simulink model "ofdm_txrx_mimo_2x"  (Simulink 6.6)
settings: Solver=VariableStepDiscrete  StartTime=0.0  StopTime=20e3  RelTol=1e-3
PreLoadFcn:
  | ofdm_rx_mimo_init
InitFcn:
  | ofdm_rx_mimo_init
StartFcn:
  | ofdm_rx_mimo_init
values from neighbouring files (symbols this model uses):
  BER_RAM_init_size = 2048    [ofdm_tx_mimo_init.m]
  CPLength = 16    [ofdm_tx_mimo_init.m]
  CRCPolynomial = hex2dec('04c11db7')    [ofdm_tx_mimo_init.m]
  freqOffset_track_filtCoef_i = hex2dec('2500')/2^32    [ofdm_rx_mimo_init.m]
  freqOffset_track_filtCoef_p = hex2dec('d0000')/2^32    [ofdm_rx_mimo_init.m]
  hi = zeros(1,length(cci))    [ofdm_rx_mimo_init.m]
  hr = zeros(1,length(ccr))    [ofdm_rx_mimo_init.m]
  max_CP_length = 16    [ofdm_tx_mimo_init.m]
  max_num_baseRateSymbols = 31    [ofdm_tx_mimo_init.m]
  max_num_subcarriers = 64    [ofdm_tx_mimo_init.m]
  max_num_trainingSymbols = 15    [ofdm_tx_mimo_init.m]
  max_numBytes = 16384    [ofdm_tx_mimo_init.m]
  max_OFDM_symbols = 2047    [ofdm_tx_mimo_init.m]
  modConstellation_qam16 = .75*[1 3 -1 -3]./3    [ofdm_tx_mimo_init.m]
  modConstellation_qam256 = 0.9375*[3 1 5 7 11 9 13 15 -3 -1 -5 -7 -11 -9 -13 -15]./15    [ofdm_tx_mimo_init.m]
  modConstellation_qam64 = 0.875*[3 1 5 7 -3 -1 -5 -7]./7    [ofdm_tx_mimo_init.m]
  modConstellation_qpsk = [1 -1]./sqrt(2)    [ofdm_tx_mimo_init.m]
  numBaseRateSymbols = 2    [ofdm_tx_mimo_init.m]
  numSubcarriers = 64    [ofdm_tx_mimo_init.m]
  numTrainingSymbols = 2    [ofdm_tx_mimo_init.m]
  pilot1_indicies = 7 + ( (64-7) * 2^16)    (= 3735559)    [ofdm_tx_mimo_init.m]
  pilot2_indicies = 21 + ( (64-21) * 2^16)    (= 2818069)    [ofdm_tx_mimo_init.m]
  pilotValue_neg = hex2dec('8000') + (2^16 * 0)    [ofdm_tx_mimo_init.m]
  pilotValue_pos = hex2dec('7FFF') + (2^16 * 0)    [ofdm_tx_mimo_init.m]
  pkt_crossCorr_thresh = 0.7    [ofdm_rx_mimo_init.m]
  pkt_energy_thresh = 0    [ofdm_rx_mimo_init.m]
  pkt_numPayloadBytes = 1400+42-6    (= 1436)    [ofdm_tx_mimo_init.m]
  pktByteNums = numHeaderBytes +  				byteIndex_numPayloadBytes(1)*2^8 +  				byteIndex_numPayloadBytes(2)*2^16 +  				byteIndex_simpleDynModMasks * 2^24    [ofdm_rx_mimo_init.m]
  PktDet_Delay = 26 + Symbol_Timing_Offset*2^7    (= 1050)    [ofdm_rx_mimo_init.m]
  preamble = 6*[0 shortsyms_10 longsyms_2]    [ofdm_tx_mimo_init.m]
  preamble_I = real(preamble)    [ofdm_tx_mimo_init.m]
  preamble_Q = imag(preamble)    [ofdm_tx_mimo_init.m]
  RAM_init_size = max_numBytes    (= 16384)    [ofdm_tx_mimo_init.m]
  reg_Interrupt_PktBuf_Control = reg_InterruptControl +  	1 * 2^16 +  	0 * 2^24    (= 65656)    [ofdm_rx_mimo_init.m]
  rx_controlBits = 1 * 2  		 + 1 * 4  		 + 0 * 8  		 + 1 * 16 * rx_SISO_Mode  		 + 1 * 32  		 + 1 * 64  		 + 0 * 128  		 + 1 * 256  		 + 0 * 512  		 + 1 * 1024  		 + 0 * 2048  		 + 1 * 4096  		 + 1 * 8192  		 + 0 * 16384  		 + 1 * 65536  		 + 1 * 131072  		 + 0 * 262144  		 + 1 * 524288    [ofdm_rx_mimo_init.m]
  Rx_PhaseNoiseTrack_K = 0.6094    [ofdm_rx_mimo_init.m]
  rx_postEq_scaling = round(rxScaling*2^11) * (1 + 2^16)    [ofdm_rx_mimo_init.m]
  RxDataScrambling_Seq = [40 198 78 63 82 173 102 245 48 111 172 115 147 230 216 93 72 65 62 2 205 242 122 90 128 83 105 97 73 10 5 252]    [ofdm_tx_mimo_init.m]
  simOnly_numSamples = length(preamble)+( (numSubcarriers+CPLength)*(numTrainingSymbols + numBaseRateSymbols + numFullRateSymbols/2) )    [ofdm_tx_mimo_init.m]
  train = [train train]    [ofdm_tx_mimo_init.m]
  tx_controlBits = (antB_preambleShift * 2^4) + (2^0 * tx_SISO_Mode) + 4*0 + 8*1    (= 73)    [ofdm_tx_mimo_init.m]
  TxDataScrambling_Seq = [40 198 78 63 82 173 102 245 48 111 172 115 147 230 216 93 72 65 62 2 205 242 122 90 128 83 105 97 73 10 5 252]    [ofdm_tx_mimo_init.m]
  txReg_symbolCounts = (2^16 * numFullRateSymbols) + (2^8 *numBaseRateSymbols ) + numTrainingSymbols    [ofdm_tx_mimo_init.m]
  TxRx_FFTScaling = bin2dec('010111') + (bin2dec('000101') * 2^6)    [ofdm_tx_mimo_init.m]
undefined symbols (not defined in the file or in its folder): AntA, AntB, BaseRate, CRC_table_gen, gcbh, intfilt, pktTiming_count_payloadStart, pktTiming_symbol_length, xlBlockLoadCallback, xlGetSimulinkPeriod
libraries: xbsIndex_r4 x3973, dspsigops x4, commchan3 x2, commrflib2 x2, dspsrcs4 x1
(part 7 of 38)

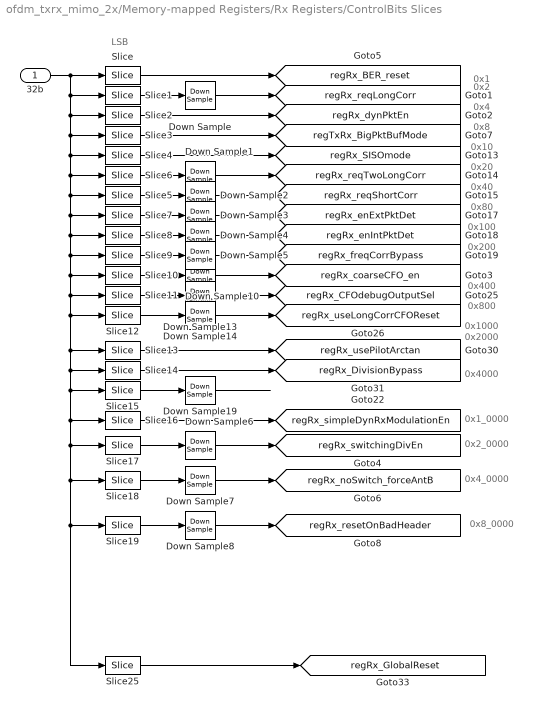
== system ofdm_txrx_mimo_2x/Memory-mapped Registers/Rx Registers/ControlBits Slices ==
  note: LSB
  note: 0x1
  note: 0x2
  note: 0x4
  note: 0x8
  note: 0x10
  note: 0x20
  note: 0x40
  note: 0x80
  note: 0x100
  note: 0x200
  note: 0x400
  note: 0x800
  note: 0x1000
  note: 0x2000
  note: 0x4000
  note: 0x1_0000
  note: 0x2_0000
  note: 0x4_0000
  note: 0x8_0000
  32b : Inport
  Down Sample{,1..8,10,13..14,19} (13 alike) : xbsIndex_r4/Down Sample  sample_ratio=2  sample_phase="Last Value of Frame  (most efficient)"  en=off  latency=1  dbl_ovrd=off  block_type=dsamp
      settings: block_version=VER_STRING_GOES_HERE
  Slice : xbsIndex_r4/Slice  nbits=1  boolean_output=on  mode=Lower Bit Location + Width  bit1=0  base1=MSB of Input  bit0=0  base0=LSB of Input  dbl_ovrd=off  block_type=slice
      settings: infoedit="Extracts a given range of bits from each input sample and presents it at the output.  The output type is ordinarily unsigned with binary point at zero, but can be Boolean when the slice is one bit wide.<P><P>Hardware notes: In hardware this block costs nothing."  block_version=VER_STRING_GOES_HERE
  Slice1 : xbsIndex_r4/Slice  nbits=1  boolean_output=on  mode=Lower Bit Location + Width  bit1=0  base1=MSB of Input  bit0=1  base0=LSB of Input  dbl_ovrd=off  block_type=slice
      settings: infoedit="Extracts a given range of bits from each input sample and presents it at the output.  The output type is ordinarily unsigned with binary point at zero, but can be Boolean when the slice is one bit wide.<P><P>Hardware notes: In hardware this block costs nothing."  block_version=VER_STRING_GOES_HERE
  Slice10 : xbsIndex_r4/Slice  nbits=1  boolean_output=on  mode=Lower Bit Location + Width  bit1=0  base1=MSB of Input  bit0=10  base0=LSB of Input  dbl_ovrd=off  block_type=slice
      settings: infoedit="Extracts a given range of bits from each input sample and presents it at the output.  The output type is ordinarily unsigned with binary point at zero, but can be Boolean when the slice is one bit wide.<P><P>Hardware notes: In hardware this block costs nothing."  block_version=VER_STRING_GOES_HERE
  Slice11 : xbsIndex_r4/Slice  nbits=1  boolean_output=on  mode=Lower Bit Location + Width  bit1=0  base1=MSB of Input  bit0=11  base0=LSB of Input  dbl_ovrd=off  block_type=slice
      settings: infoedit="Extracts a given range of bits from each input sample and presents it at the output.  The output type is ordinarily unsigned with binary point at zero, but can be Boolean when the slice is one bit wide.<P><P>Hardware notes: In hardware this block costs nothing."  block_version=VER_STRING_GOES_HERE
  Slice12 : xbsIndex_r4/Slice  nbits=1  boolean_output=on  mode=Lower Bit Location + Width  bit1=0  base1=MSB of Input  bit0=12  base0=LSB of Input  dbl_ovrd=off  block_type=slice
      settings: infoedit="Extracts a given range of bits from each input sample and presents it at the output.  The output type is ordinarily unsigned with binary point at zero, but can be Boolean when the slice is one bit wide.<P><P>Hardware notes: In hardware this block costs nothing."  block_version=VER_STRING_GOES_HERE
  Slice13 : xbsIndex_r4/Slice  nbits=1  boolean_output=on  mode=Lower Bit Location + Width  bit1=0  base1=MSB of Input  bit0=13  base0=LSB of Input  dbl_ovrd=off  block_type=slice
      settings: infoedit="Extracts a given range of bits from each input sample and presents it at the output.  The output type is ordinarily unsigned with binary point at zero, but can be Boolean when the slice is one bit wide.<P><P>Hardware notes: In hardware this block costs nothing."  block_version=VER_STRING_GOES_HERE
  Slice14 : xbsIndex_r4/Slice  nbits=1  boolean_output=on  mode=Lower Bit Location + Width  bit1=0  base1=MSB of Input  bit0=14  base0=LSB of Input  dbl_ovrd=off  block_type=slice
      settings: infoedit="Extracts a given range of bits from each input sample and presents it at the output.  The output type is ordinarily unsigned with binary point at zero, but can be Boolean when the slice is one bit wide.<P><P>Hardware notes: In hardware this block costs nothing."  block_version=VER_STRING_GOES_HERE
  Slice15 : xbsIndex_r4/Slice  nbits=1  boolean_output=on  mode=Lower Bit Location + Width  bit1=0  base1=MSB of Input  bit0=15  base0=LSB of Input  dbl_ovrd=off  block_type=slice
      settings: infoedit="Extracts a given range of bits from each input sample and presents it at the output.  The output type is ordinarily unsigned with binary point at zero, but can be Boolean when the slice is one bit wide.<P><P>Hardware notes: In hardware this block costs nothing."  block_version=VER_STRING_GOES_HERE
  Slice16 : xbsIndex_r4/Slice  nbits=1  boolean_output=on  mode=Lower Bit Location + Width  bit1=0  base1=MSB of Input  bit0=16  base0=LSB of Input  dbl_ovrd=off  block_type=slice
      settings: infoedit="Extracts a given range of bits from each input sample and presents it at the output.  The output type is ordinarily unsigned with binary point at zero, but can be Boolean when the slice is one bit wide.<P><P>Hardware notes: In hardware this block costs nothing."  block_version=VER_STRING_GOES_HERE
  Slice17 : xbsIndex_r4/Slice  nbits=1  boolean_output=on  mode=Lower Bit Location + Width  bit1=0  base1=MSB of Input  bit0=17  base0=LSB of Input  dbl_ovrd=off  block_type=slice
      settings: infoedit="Extracts a given range of bits from each input sample and presents it at the output.  The output type is ordinarily unsigned with binary point at zero, but can be Boolean when the slice is one bit wide.<P><P>Hardware notes: In hardware this block costs nothing."  block_version=VER_STRING_GOES_HERE
  Slice18 : xbsIndex_r4/Slice  nbits=1  boolean_output=on  mode=Lower Bit Location + Width  bit1=0  base1=MSB of Input  bit0=18  base0=LSB of Input  dbl_ovrd=off  block_type=slice
      settings: infoedit="Extracts a given range of bits from each input sample and presents it at the output.  The output type is ordinarily unsigned with binary point at zero, but can be Boolean when the slice is one bit wide.<P><P>Hardware notes: In hardware this block costs nothing."  block_version=VER_STRING_GOES_HERE
  Slice19 : xbsIndex_r4/Slice  nbits=1  boolean_output=on  mode=Lower Bit Location + Width  bit1=0  base1=MSB of Input  bit0=19  base0=LSB of Input  dbl_ovrd=off  block_type=slice
      settings: infoedit="Extracts a given range of bits from each input sample and presents it at the output.  The output type is ordinarily unsigned with binary point at zero, but can be Boolean when the slice is one bit wide.<P><P>Hardware notes: In hardware this block costs nothing."  block_version=VER_STRING_GOES_HERE
  Slice2 : xbsIndex_r4/Slice  nbits=1  boolean_output=on  mode=Lower Bit Location + Width  bit1=0  base1=MSB of Input  bit0=2  base0=LSB of Input  dbl_ovrd=off  block_type=slice
      settings: infoedit="Extracts a given range of bits from each input sample and presents it at the output.  The output type is ordinarily unsigned with binary point at zero, but can be Boolean when the slice is one bit wide.<P><P>Hardware notes: In hardware this block costs nothing."  block_version=VER_STRING_GOES_HERE
  Slice25 : xbsIndex_r4/Slice  nbits=1  boolean_output=on  mode=Upper Bit Location + Width  bit1=0  base1=MSB of Input  bit0=31  base0=LSB of Input  dbl_ovrd=off  block_type=slice
      settings: infoedit="Extracts a given range of bits from each input sample and presents it at the output.  The output type is ordinarily unsigned with binary point at zero, but can be Boolean when the slice is one bit wide.<P><P>Hardware notes: In hardware this block costs nothing."  block_version=VER_STRING_GOES_HERE
  Slice3 : xbsIndex_r4/Slice  nbits=1  boolean_output=on  mode=Lower Bit Location + Width  bit1=0  base1=MSB of Input  bit0=3  base0=LSB of Input  dbl_ovrd=off  block_type=slice
      settings: infoedit="Extracts a given range of bits from each input sample and presents it at the output.  The output type is ordinarily unsigned with binary point at zero, but can be Boolean when the slice is one bit wide.<P><P>Hardware notes: In hardware this block costs nothing."  block_version=VER_STRING_GOES_HERE
  Slice4 : xbsIndex_r4/Slice  nbits=1  boolean_output=on  mode=Lower Bit Location + Width  bit1=0  base1=MSB of Input  bit0=4  base0=LSB of Input  dbl_ovrd=off  block_type=slice
      settings: infoedit="Extracts a given range of bits from each input sample and presents it at the output.  The output type is ordinarily unsigned with binary point at zero, but can be Boolean when the slice is one bit wide.<P><P>Hardware notes: In hardware this block costs nothing."  block_version=VER_STRING_GOES_HERE
  Slice5 : xbsIndex_r4/Slice  nbits=1  boolean_output=on  mode=Lower Bit Location + Width  bit1=0  base1=MSB of Input  bit0=6  base0=LSB of Input  dbl_ovrd=off  block_type=slice
      settings: infoedit="Extracts a given range of bits from each input sample and presents it at the output.  The output type is ordinarily unsigned with binary point at zero, but can be Boolean when the slice is one bit wide.<P><P>Hardware notes: In hardware this block costs nothing."  block_version=VER_STRING_GOES_HERE
  Slice6 : xbsIndex_r4/Slice  nbits=1  boolean_output=on  mode=Lower Bit Location + Width  bit1=0  base1=MSB of Input  bit0=5  base0=LSB of Input  dbl_ovrd=off  block_type=slice
      settings: infoedit="Extracts a given range of bits from each input sample and presents it at the output.  The output type is ordinarily unsigned with binary point at zero, but can be Boolean when the slice is one bit wide.<P><P>Hardware notes: In hardware this block costs nothing."  block_version=VER_STRING_GOES_HERE
  Slice7 : xbsIndex_r4/Slice  nbits=1  boolean_output=on  mode=Lower Bit Location + Width  bit1=0  base1=MSB of Input  bit0=7  base0=LSB of Input  dbl_ovrd=off  block_type=slice
      settings: infoedit="Extracts a given range of bits from each input sample and presents it at the output.  The output type is ordinarily unsigned with binary point at zero, but can be Boolean when the slice is one bit wide.<P><P>Hardware notes: In hardware this block costs nothing."  block_version=VER_STRING_GOES_HERE
  Slice8 : xbsIndex_r4/Slice  nbits=1  boolean_output=on  mode=Lower Bit Location + Width  bit1=0  base1=MSB of Input  bit0=8  base0=LSB of Input  dbl_ovrd=off  block_type=slice
      settings: infoedit="Extracts a given range of bits from each input sample and presents it at the output.  The output type is ordinarily unsigned with binary point at zero, but can be Boolean when the slice is one bit wide.<P><P>Hardware notes: In hardware this block costs nothing."  block_version=VER_STRING_GOES_HERE
  Slice9 : xbsIndex_r4/Slice  nbits=1  boolean_output=on  mode=Lower Bit Location + Width  bit1=0  base1=MSB of Input  bit0=9  base0=LSB of Input  dbl_ovrd=off  block_type=slice
      settings: infoedit="Extracts a given range of bits from each input sample and presents it at the output.  The output type is ordinarily unsigned with binary point at zero, but can be Boolean when the slice is one bit wide.<P><P>Hardware notes: In hardware this block costs nothing."  block_version=VER_STRING_GOES_HERE
  tag scope: regRx_BER_reset=global, regRx_CFOdebugOutputSel=global, regRx_DivisionBypass=global, regRx_GlobalReset=global, regRx_SISOmode=global, regRx_coarseCFO_en=global, regRx_dynPktEn=global, regRx_enExtPktDet=global, regRx_enIntPktDet=global, regRx_freqCorrBypass=global, regRx_noSwitch_forceAntB=global, regRx_reqLongCorr=global, regRx_reqShortCorr=global, regRx_reqTwoLongCorr=global, regRx_resetOnBadHeader=global, regRx_simpleDynRxModulationEn=global, regRx_switchingDivEn=global, regRx_useLongCorrCFOReset=global, regRx_usePilotArctan=global, regTxRx_BigPktBufMode=global
  wires:
  Slice/1 -> [regRx_BER_reset]
  Slice1/1 -> Down Sample/1
  Slice2/1 -> [regRx_dynPktEn]
  Down Sample/1 -> [regRx_reqLongCorr]
  Slice4/1 -> [regRx_SISOmode]
  Slice6/1 -> Down Sample1/1
  Slice5/1 -> Down Sample2/1
  Down Sample1/1 -> [regRx_reqTwoLongCorr]
  Down Sample2/1 -> [regRx_reqShortCorr]
  Slice7/1 -> Down Sample3/1
  Slice8/1 -> Down Sample4/1
  Down Sample3/1 -> [regRx_enExtPktDet]
  Down Sample4/1 -> [regRx_enIntPktDet]
  Slice9/1 -> Down Sample5/1
  Down Sample5/1 -> [regRx_freqCorrBypass]
  32b/1 -> Slice16/1, Slice17/1, Slice18/1, Slice25/1, Slice19/1, Slice15/1, Slice14/1, Slice13/1, Slice12/1, Slice11/1, Slice10/1, Slice9/1, Slice8/1, Slice7/1, Slice5/1, Slice6/1, Slice4/1, Slice3/1, Slice2/1, Slice1/1, Slice/1
  Slice10/1 -> Down Sample10/1
  Down Sample10/1 -> [regRx_coarseCFO_en]
  Slice11/1 -> Down Sample13/1
  Down Sample13/1 -> [regRx_CFOdebugOutputSel]
  Down Sample14/1 -> [regRx_useLongCorrCFOReset]
  Slice12/1 -> Down Sample14/1
  Slice3/1 -> [regTxRx_BigPktBufMode]
  Slice13/1 -> [regRx_usePilotArctan]
  Slice14/1 -> [regRx_DivisionBypass]
  Slice15/1 -> Down Sample19/1
  Down Sample19/1 -> ?
  Slice25/1 -> [regRx_GlobalReset]
  Slice16/1 -> [regRx_simpleDynRxModulationEn]
  Slice17/1 -> Down Sample6/1
  Down Sample6/1 -> [regRx_switchingDivEn]
  Slice18/1 -> Down Sample7/1
  Down Sample7/1 -> [regRx_noSwitch_forceAntB]
  Down Sample8/1 -> [regRx_resetOnBadHeader]
  Slice19/1 -> Down Sample8/1

== system ofdm_txrx_mimo_2x/Memory-mapped Registers/Rx Registers/Read Only Register ==
  D : Inport
  En : Inport  Port=2
  Convert : xbsIndex_r4/Convert  arith_type=Unsigned  n_bits=32  bin_pt=0  quantization=Truncate  overflow=Wrap  latency=0  dbl_ovrd=off  pipeline=off  block_type=convert
      settings: block_version=10.1
  Reinterpret : xbsIndex_r4/Reinterpret  force_arith_type=on  arith_type=Unsigned  force_bin_pt=on  bin_pt=0  block_type=reinterpret
      settings: infoedit="Changes signal type without altering the binary representation.   You can changed the signal between signed and unsigned, and relocate the binary point.<P><P>Hardware notes: In hardware this block costs nothing.<P><P>Example:  Suppose the input is 6 bits wide, signed, with 2 fractional bits, and the output is forced to unsigned with 0 fractional bits.  Then an input of -2.0 (1110.00 in binary 2's complement) becomes an output of 56 (111000 in binary)."  block_version=10.1
  Terminator : Terminator
  To Register : xbsIndex_r4/To Register  shared_memory_name='Rx_Gains'  init=0  ownership=Locally owned and initialized  explicit_data_type=on  arith_type=Unsigned  n_bits=32  bin_pt=0  dbl_ovrd=off  block_type=toreg
      settings: AttributesFormatString=To Register\n<< %<shared_memory_name> >>  infoedit="Register block that writes data to a shared memory register.  Delay of one sample period."  block_version=8.2.02
  wires:
  En/1 -> To Register/2
  To Register/1 -> Terminator/1
  D/1 -> Reinterpret/1
  Reinterpret/1 -> Convert/1
  Convert/1 -> To Register/1

== system ofdm_txrx_mimo_2x/Memory-mapped Registers/Rx Registers/Read Only Register1 ==
  D : Inport
  En : Inport  Port=2
  Convert : xbsIndex_r4/Convert  arith_type=Unsigned  n_bits=32  bin_pt=0  quantization=Truncate  overflow=Wrap  latency=0  dbl_ovrd=off  pipeline=off  block_type=convert
      settings: block_version=10.1
  Reinterpret : xbsIndex_r4/Reinterpret  force_arith_type=on  arith_type=Unsigned  force_bin_pt=on  bin_pt=0  block_type=reinterpret
      settings: infoedit="Changes signal type without altering the binary representation.   You can changed the signal between signed and unsigned, and relocate the binary point.<P><P>Hardware notes: In hardware this block costs nothing.<P><P>Example:  Suppose the input is 6 bits wide, signed, with 2 fractional bits, and the output is forced to unsigned with 0 fractional bits.  Then an input of -2.0 (1110.00 in binary 2's complement) becomes an output of 56 (111000 in binary)."  block_version=10.1
  Terminator : Terminator
  To Register : xbsIndex_r4/To Register  shared_memory_name='Rx_BER_Errors'  init=0  ownership=Locally owned and initialized  explicit_data_type=on  arith_type=Unsigned  n_bits=32  bin_pt=0  dbl_ovrd=off  block_type=toreg
      settings: AttributesFormatString=To Register\n<< %<shared_memory_name> >>  infoedit="Register block that writes data to a shared memory register.  Delay of one sample period."  block_version=8.2.02
  wires:
  En/1 -> To Register/2
  To Register/1 -> Terminator/1
  D/1 -> Reinterpret/1
  Reinterpret/1 -> Convert/1
  Convert/1 -> To Register/1

== system ofdm_txrx_mimo_2x/Memory-mapped Registers/Rx Registers/Read Only Register2 ==
  D : Inport
  En : Inport  Port=2
  Convert : xbsIndex_r4/Convert  arith_type=Unsigned  n_bits=32  bin_pt=0  quantization=Truncate  overflow=Wrap  latency=0  dbl_ovrd=off  pipeline=off  block_type=convert
      settings: block_version=10.1
  Reinterpret : xbsIndex_r4/Reinterpret  force_arith_type=on  arith_type=Unsigned  force_bin_pt=on  bin_pt=0  block_type=reinterpret
      settings: infoedit="Changes signal type without altering the binary representation.   You can changed the signal between signed and unsigned, and relocate the binary point.<P><P>Hardware notes: In hardware this block costs nothing.<P><P>Example:  Suppose the input is 6 bits wide, signed, with 2 fractional bits, and the output is forced to unsigned with 0 fractional bits.  Then an input of -2.0 (1110.00 in binary 2's complement) becomes an output of 56 (111000 in binary)."  block_version=10.1
  Terminator : Terminator
  To Register : xbsIndex_r4/To Register  shared_memory_name='Rx_BER_TotalBits'  init=0  ownership=Locally owned and initialized  explicit_data_type=on  arith_type=Unsigned  n_bits=32  bin_pt=0  dbl_ovrd=off  block_type=toreg
      settings: AttributesFormatString=To Register\n<< %<shared_memory_name> >>  infoedit="Register block that writes data to a shared memory register.  Delay of one sample period."  block_version=8.2.02
  wires:
  En/1 -> To Register/2
  To Register/1 -> Terminator/1
  D/1 -> Reinterpret/1
  Reinterpret/1 -> Convert/1
  Convert/1 -> To Register/1

== system ofdm_txrx_mimo_2x/Memory-mapped Registers/Rx Registers/Read Only Register3 ==
  D : Inport
  En : Inport  Port=2
  Convert : xbsIndex_r4/Convert  arith_type=Unsigned  n_bits=32  bin_pt=0  quantization=Truncate  overflow=Wrap  latency=0  dbl_ovrd=off  pipeline=off  block_type=convert
      settings: block_version=10.1
  Reinterpret : xbsIndex_r4/Reinterpret  force_arith_type=on  arith_type=Unsigned  force_bin_pt=on  bin_pt=0  block_type=reinterpret
      settings: infoedit="Changes signal type without altering the binary representation.   You can changed the signal between signed and unsigned, and relocate the binary point.<P><P>Hardware notes: In hardware this block costs nothing.<P><P>Example:  Suppose the input is 6 bits wide, signed, with 2 fractional bits, and the output is forced to unsigned with 0 fractional bits.  Then an input of -2.0 (1110.00 in binary 2's complement) becomes an output of 56 (111000 in binary)."  block_version=10.1
  Terminator : Terminator
  To Register : xbsIndex_r4/To Register  shared_memory_name='Rx_pktDone_interruptStatus'  init=0  ownership=Locally owned and initialized  explicit_data_type=on  arith_type=Unsigned  n_bits=32  bin_pt=0  dbl_ovrd=off  block_type=toreg
      settings: AttributesFormatString=To Register\n<< %<shared_memory_name> >>  infoedit="Register block that writes data to a shared memory register.  Delay of one sample period."  block_version=8.2.02
  wires:
  En/1 -> To Register/2
  To Register/1 -> Terminator/1
  D/1 -> Reinterpret/1
  Reinterpret/1 -> Convert/1
  Convert/1 -> To Register/1

== system ofdm_txrx_mimo_2x/Memory-mapped Registers/Rx Registers/Read-Write Register ==
  Constant : xbsIndex_r4/Constant  arith_type=Boolean  const=1  n_bits=16  bin_pt=14  explicit_period=on  period=sampPeriod  equ=P=C  opselect=C  inp2=PCIN>>17  opr=+  inp1=P  carry=CIN  dbl_ovrd=off  block_type=constant
      settings: block_version=8.2.02
  From Register : xbsIndex_r4/From Register  shared_memory_name='Rx_ControlBits_w'  init=734566  period=sampPeriod  ownership=Locally owned and initialized  arith_type=Unsigned  n_bits=32  bin_pt=0  dbl_ovrd=off  block_type=fromreg
      settings: AttributesFormatString=From Register\n<< %<shared_memory_name> >>\n%<n_bits>\n%<period>\n%<bin_pt>\n%<arith_type>\n%<ownership>\n%<init>  infoedit="Register block that reads data to a shared memory register.  Delay of one sample period."  block_version=8.2.02
  Terminator : Terminator
  To Register : xbsIndex_r4/To Register  shared_memory_name='Rx_ControlBits_r'  init=734566  ownership=Locally owned and initialized  explicit_data_type=on  arith_type=Unsigned  n_bits=32  bin_pt=0  dbl_ovrd=off  block_type=toreg
      settings: AttributesFormatString=To Register\n<< %<shared_memory_name> >>  infoedit="Register block that writes data to a shared memory register.  Delay of one sample period."  block_version=8.2.02
  D : Outport
  wires:
  To Register/1 -> Terminator/1
  Constant/1 -> To Register/2
  From Register/1 -> To Register/1, D/1

== system ofdm_txrx_mimo_2x/Memory-mapped Registers/Rx Registers/Read-Write Register1 ==
  Constant : xbsIndex_r4/Constant  arith_type=Boolean  const=1  n_bits=16  bin_pt=14  explicit_period=on  period=sampPeriod  equ=P=C  opselect=C  inp2=PCIN>>17  opr=+  inp1=P  carry=CIN  dbl_ovrd=off  block_type=constant
      settings: block_version=8.2.02
  From Register : xbsIndex_r4/From Register  shared_memory_name='Rx_PktDet_LongCorr_Params_w'  init=196608235  period=sampPeriod  ownership=Locally owned and initialized  arith_type=Unsigned  n_bits=32  bin_pt=0  dbl_ovrd=off  block_type=fromreg
      settings: AttributesFormatString=From Register\n<< %<shared_memory_name> >>\n%<n_bits>\n%<period>\n%<bin_pt>\n%<arith_type>\n%<ownership>\n%<init>  infoedit="Register block that reads data to a shared memory register.  Delay of one sample period."  block_version=8.2.02
  Terminator : Terminator
  To Register : xbsIndex_r4/To Register  shared_memory_name='Rx_PktDet_LongCorr_Params_r'  init=196608235  ownership=Locally owned and initialized  explicit_data_type=on  arith_type=Unsigned  n_bits=32  bin_pt=0  dbl_ovrd=off  block_type=toreg
      settings: AttributesFormatString=To Register\n<< %<shared_memory_name> >>  infoedit="Register block that writes data to a shared memory register.  Delay of one sample period."  block_version=8.2.02
  D : Outport
  wires:
  To Register/1 -> Terminator/1
  Constant/1 -> To Register/2
  From Register/1 -> To Register/1, D/1

== system ofdm_txrx_mimo_2x/Memory-mapped Registers/Rx Registers/Read-Write Register2 ==
  Constant : xbsIndex_r4/Constant  arith_type=Boolean  const=1  n_bits=16  bin_pt=14  explicit_period=on  period=sampPeriod  equ=P=C  opselect=C  inp2=PCIN>>17  opr=+  inp1=P  carry=CIN  dbl_ovrd=off  block_type=constant
      settings: block_version=8.2.02
  From Register : xbsIndex_r4/From Register  shared_memory_name='Rx_pktByteNums_w'  init=131864  period=sampPeriod  ownership=Locally owned and initialized  arith_type=Unsigned  n_bits=32  bin_pt=0  dbl_ovrd=off  block_type=fromreg
      settings: AttributesFormatString=From Register\n<< %<shared_memory_name> >>\n%<n_bits>\n%<period>\n%<bin_pt>\n%<arith_type>\n%<ownership>\n%<init>  infoedit="Register block that reads data to a shared memory register.  Delay of one sample period."  block_version=8.2.02
  Terminator : Terminator
  To Register : xbsIndex_r4/To Register  shared_memory_name='Rx_pktByteNums_r'  init=131864  ownership=Locally owned and initialized  explicit_data_type=on  arith_type=Unsigned  n_bits=32  bin_pt=0  dbl_ovrd=off  block_type=toreg
      settings: AttributesFormatString=To Register\n<< %<shared_memory_name> >>  infoedit="Register block that writes data to a shared memory register.  Delay of one sample period."  block_version=8.2.02
  D : Outport
  wires:
  To Register/1 -> Terminator/1
  Constant/1 -> To Register/2
  From Register/1 -> To Register/1, D/1

== system ofdm_txrx_mimo_2x/Memory-mapped Registers/Rx Registers/Read-Write Register3 ==
  Constant : xbsIndex_r4/Constant  arith_type=Boolean  const=1  n_bits=16  bin_pt=14  explicit_period=on  period=sampPeriod  equ=P=C  opselect=C  inp2=PCIN>>17  opr=+  inp1=P  carry=CIN  dbl_ovrd=off  block_type=constant
      settings: block_version=8.2.02
  From Register : xbsIndex_r4/From Register  shared_memory_name='Rx_PktDet_Delay_w'  init=1050  period=sampPeriod  ownership=Locally owned and initialized  arith_type=Unsigned  n_bits=32  bin_pt=0  dbl_ovrd=off  block_type=fromreg
      settings: AttributesFormatString=From Register\n<< %<shared_memory_name> >>\n%<n_bits>\n%<period>\n%<bin_pt>\n%<arith_type>\n%<ownership>\n%<init>  infoedit="Register block that reads data to a shared memory register.  Delay of one sample period."  block_version=8.2.02
  Terminator : Terminator
  To Register : xbsIndex_r4/To Register  shared_memory_name='Rx_PktDet_Delay_r'  init=1050  ownership=Locally owned and initialized  explicit_data_type=on  arith_type=Unsigned  n_bits=32  bin_pt=0  dbl_ovrd=off  block_type=toreg
      settings: AttributesFormatString=To Register\n<< %<shared_memory_name> >>  infoedit="Register block that writes data to a shared memory register.  Delay of one sample period."  block_version=8.2.02
  D : Outport
  wires:
  To Register/1 -> Terminator/1
  Constant/1 -> To Register/2
  From Register/1 -> To Register/1, D/1

== system ofdm_txrx_mimo_2x/Memory-mapped Registers/Rx Registers/Read-Write Register4 ==
  Constant : xbsIndex_r4/Constant  arith_type=Boolean  const=1  n_bits=16  bin_pt=14  explicit_period=on  period=sampPeriod  equ=P=C  opselect=C  inp2=PCIN>>17  opr=+  inp1=P  carry=CIN  dbl_ovrd=off  block_type=constant
      settings: block_version=8.2.02
  From Register : xbsIndex_r4/From Register  shared_memory_name='Rx_OFDM_SymbolCounts_w'  init=131074  period=sampPeriod  ownership=Locally owned and initialized  arith_type=Unsigned  n_bits=32  bin_pt=0  dbl_ovrd=off  block_type=fromreg
      settings: AttributesFormatString=From Register\n<< %<shared_memory_name> >>\n%<n_bits>\n%<period>\n%<bin_pt>\n%<arith_type>\n%<ownership>\n%<init>  infoedit="Register block that reads data to a shared memory register.  Delay of one sample period."  block_version=8.2.02
  Terminator : Terminator
  To Register : xbsIndex_r4/To Register  shared_memory_name='Rx_OFDM_SymbolCounts_r'  init=131074  ownership=Locally owned and initialized  explicit_data_type=on  arith_type=Unsigned  n_bits=32  bin_pt=0  dbl_ovrd=off  block_type=toreg
      settings: AttributesFormatString=To Register\n<< %<shared_memory_name> >>  infoedit="Register block that writes data to a shared memory register.  Delay of one sample period."  block_version=8.2.02
  D : Outport
  wires:
  To Register/1 -> Terminator/1
  Constant/1 -> To Register/2
  From Register/1 -> To Register/1, D/1

== system ofdm_txrx_mimo_2x/Memory-mapped Registers/Rx Registers/Write-Only Register ==
  From Register : xbsIndex_r4/From Register  shared_memory_name='Rx_pktDet_Tresholds_w'  init=0.7  period=sampPeriod  ownership=Locally owned and initialized  arith_type=Unsigned  n_bits=32  bin_pt=0  dbl_ovrd=off  block_type=fromreg
      settings: AttributesFormatString=From Register\n<< %<shared_memory_name> >>\n%<n_bits>\n%<period>\n%<bin_pt>\n%<arith_type>\n%<ownership>\n%<init>  infoedit="Register block that reads data to a shared memory register.  Delay of one sample period."  block_version=8.2.02
  D : Outport
  wires:
  From Register/1 -> D/1

== system ofdm_txrx_mimo_2x/Memory-mapped Registers/Rx Registers/Write-Only Register1 ==
  From Register : xbsIndex_r4/From Register  shared_memory_name='Rx_Constellation_Scaling_w'  init=268439552  period=sampPeriod  ownership=Locally owned and initialized  arith_type=Unsigned  n_bits=32  bin_pt=0  dbl_ovrd=off  block_type=fromreg
      settings: AttributesFormatString=From Register\n<< %<shared_memory_name> >>\n%<n_bits>\n%<period>\n%<bin_pt>\n%<arith_type>\n%<ownership>\n%<init>  infoedit="Register block that reads data to a shared memory register.  Delay of one sample period."  block_version=8.2.02
  D : Outport
  wires:
  From Register/1 -> D/1

== system ofdm_txrx_mimo_2x/Memory-mapped Registers/Rx Registers/Write-Only Register2 ==
  From Register : xbsIndex_r4/From Register  shared_memory_name='Rx_FreqOffFilt_B_KI_w'  init=9472  period=sampPeriod  ownership=Locally owned and initialized  arith_type=Unsigned  n_bits=32  bin_pt=0  dbl_ovrd=off  block_type=fromreg
      settings: AttributesFormatString=From Register\n<< %<shared_memory_name> >>\n%<n_bits>\n%<period>\n%<bin_pt>\n%<arith_type>\n%<ownership>\n%<init>  infoedit="Register block that reads data to a shared memory register.  Delay of one sample period."  block_version=8.2.02
  D : Outport
  wires:
  From Register/1 -> D/1

== system ofdm_txrx_mimo_2x/Memory-mapped Registers/Rx Registers/Write-Only Register3 ==
  From Register : xbsIndex_r4/From Register  shared_memory_name='Rx_FreqOffFilt_B_KP_w'  init=851968  period=sampPeriod  ownership=Locally owned and initialized  arith_type=Unsigned  n_bits=32  bin_pt=0  dbl_ovrd=off  block_type=fromreg
      settings: AttributesFormatString=From Register\n<< %<shared_memory_name> >>\n%<n_bits>\n%<period>\n%<bin_pt>\n%<arith_type>\n%<ownership>\n%<init>  infoedit="Register block that reads data to a shared memory register.  Delay of one sample period."  block_version=8.2.02
  D : Outport
  wires:
  From Register/1 -> D/1

== system ofdm_txrx_mimo_2x/Memory-mapped Registers/Rx Registers/Write-Only Register4 ==
  From Register : xbsIndex_r4/From Register  shared_memory_name='Rx_PhaseNoiseTrack_K_w'  init=40896141.7216  period=sampPeriod  ownership=Locally owned and initialized  arith_type=Unsigned  n_bits=32  bin_pt=0  dbl_ovrd=off  block_type=fromreg
      settings: AttributesFormatString=From Register\n<< %<shared_memory_name> >>\n%<n_bits>\n%<period>\n%<bin_pt>\n%<arith_type>\n%<ownership>\n%<init>  infoedit="Register block that reads data to a shared memory register.  Delay of one sample period."  block_version=8.2.02
  D : Outport
  wires:
  From Register/1 -> D/1

== system ofdm_txrx_mimo_2x/Memory-mapped Registers/Tx Registers/Read Only Register ==
  D : Inport
  En : Inport  Port=2
  Convert : xbsIndex_r4/Convert  arith_type=Unsigned  n_bits=32  bin_pt=0  quantization=Truncate  overflow=Wrap  latency=0  dbl_ovrd=off  pipeline=off  block_type=convert
      settings: block_version=10.1
  Reinterpret : xbsIndex_r4/Reinterpret  force_arith_type=on  arith_type=Unsigned  force_bin_pt=on  bin_pt=0  block_type=reinterpret
      settings: infoedit="Changes signal type without altering the binary representation.   You can changed the signal between signed and unsigned, and relocate the binary point.<P><P>Hardware notes: In hardware this block costs nothing.<P><P>Example:  Suppose the input is 6 bits wide, signed, with 2 fractional bits, and the output is forced to unsigned with 0 fractional bits.  Then an input of -2.0 (1110.00 in binary 2's complement) becomes an output of 56 (111000 in binary)."  block_version=10.1
  Terminator : Terminator
  To Register : xbsIndex_r4/To Register  shared_memory_name='Tx_PktRunning'  init=0  ownership=Locally owned and initialized  explicit_data_type=on  arith_type=Unsigned  n_bits=32  bin_pt=0  dbl_ovrd=off  block_type=toreg
      settings: AttributesFormatString=To Register\n<< %<shared_memory_name> >>  infoedit="Register block that writes data to a shared memory register.  Delay of one sample period."  block_version=8.2.02
  wires:
  En/1 -> To Register/2
  To Register/1 -> Terminator/1
  D/1 -> Reinterpret/1
  Reinterpret/1 -> Convert/1
  Convert/1 -> To Register/1

== system ofdm_txrx_mimo_2x/Memory-mapped Registers/Tx Registers/Read-Write Register ==
  Constant : xbsIndex_r4/Constant  arith_type=Boolean  const=1  n_bits=16  bin_pt=14  explicit_period=on  period=sampPeriod  equ=P=C  opselect=C  inp2=PCIN>>17  opr=+  inp1=P  carry=CIN  dbl_ovrd=off  block_type=constant
      settings: block_version=8.2.02
  From Register : xbsIndex_r4/From Register  shared_memory_name='Tx_Start_Reset_Control_w'  init=0  period=sampPeriod  ownership=Locally owned and initialized  arith_type=Unsigned  n_bits=32  bin_pt=0  dbl_ovrd=off  block_type=fromreg
      settings: AttributesFormatString=From Register\n<< %<shared_memory_name> >>\n%<n_bits>\n%<period>\n%<bin_pt>\n%<arith_type>\n%<ownership>\n%<init>  infoedit="Register block that reads data to a shared memory register.  Delay of one sample period."  block_version=8.2.02
  Terminator : Terminator
  To Register : xbsIndex_r4/To Register  shared_memory_name='Tx_Start_Reset_Control_r'  init=0  ownership=Locally owned and initialized  explicit_data_type=on  arith_type=Unsigned  n_bits=32  bin_pt=0  dbl_ovrd=off  block_type=toreg
      settings: AttributesFormatString=To Register\n<< %<shared_memory_name> >>  infoedit="Register block that writes data to a shared memory register.  Delay of one sample period."  block_version=8.2.02
  D : Outport
  wires:
  To Register/1 -> Terminator/1
  Constant/1 -> To Register/2
  From Register/1 -> To Register/1, D/1

== system ofdm_txrx_mimo_2x/Memory-mapped Registers/Tx Registers/Read-Write Register1 ==
  Constant : xbsIndex_r4/Constant  arith_type=Boolean  const=1  n_bits=16  bin_pt=14  explicit_period=on  period=sampPeriod  equ=P=C  opselect=C  inp2=PCIN>>17  opr=+  inp1=P  carry=CIN  dbl_ovrd=off  block_type=constant
      settings: block_version=8.2.02
  From Register : xbsIndex_r4/From Register  shared_memory_name='Tx_ControlBits_w'  init=72  period=sampPeriod  ownership=Locally owned and initialized  arith_type=Unsigned  n_bits=32  bin_pt=0  dbl_ovrd=off  block_type=fromreg
      settings: AttributesFormatString=From Register\n<< %<shared_memory_name> >>\n%<n_bits>\n%<period>\n%<bin_pt>\n%<arith_type>\n%<ownership>\n%<init>  infoedit="Register block that reads data to a shared memory register.  Delay of one sample period."  block_version=8.2.02
  Terminator : Terminator
  To Register : xbsIndex_r4/To Register  shared_memory_name='Tx_ControlBits_r'  init=72  ownership=Locally owned and initialized  explicit_data_type=on  arith_type=Unsigned  n_bits=32  bin_pt=0  dbl_ovrd=off  block_type=toreg
      settings: AttributesFormatString=To Register\n<< %<shared_memory_name> >>  infoedit="Register block that writes data to a shared memory register.  Delay of one sample period."  block_version=8.2.02
  D : Outport
  wires:
  To Register/1 -> Terminator/1
  Constant/1 -> To Register/2
  From Register/1 -> To Register/1, D/1

== system ofdm_txrx_mimo_2x/Memory-mapped Registers/Tx Registers/Read-Write Register2 ==
  Constant : xbsIndex_r4/Constant  arith_type=Boolean  const=1  n_bits=16  bin_pt=14  explicit_period=on  period=sampPeriod  equ=P=C  opselect=C  inp2=PCIN>>17  opr=+  inp1=P  carry=CIN  dbl_ovrd=off  block_type=constant
      settings: block_version=8.2.02
  From Register : xbsIndex_r4/From Register  shared_memory_name='Tx_OFDM_SymCounts_w'  init=7864834  period=sampPeriod  ownership=Locally owned and initialized  arith_type=Unsigned  n_bits=32  bin_pt=0  dbl_ovrd=off  block_type=fromreg
      settings: AttributesFormatString=From Register\n<< %<shared_memory_name> >>\n%<n_bits>\n%<period>\n%<bin_pt>\n%<arith_type>\n%<ownership>\n%<init>  infoedit="Register block that reads data to a shared memory register.  Delay of one sample period."  block_version=8.2.02
  Terminator : Terminator
  To Register : xbsIndex_r4/To Register  shared_memory_name='Tx_OFDM_SymCounts_r'  init=7864834  ownership=Locally owned and initialized  explicit_data_type=on  arith_type=Unsigned  n_bits=32  bin_pt=0  dbl_ovrd=off  block_type=toreg
      settings: AttributesFormatString=To Register\n<< %<shared_memory_name> >>  infoedit="Register block that writes data to a shared memory register.  Delay of one sample period."  block_version=8.2.02
  D : Outport
  wires:
  To Register/1 -> Terminator/1
  Constant/1 -> To Register/2
  From Register/1 -> To Register/1, D/1

== system ofdm_txrx_mimo_2x/Memory-mapped Registers/Tx Registers/Write-Only Register ==
  From Register : xbsIndex_r4/From Register  shared_memory_name='Tx_PreambleMasking_w'  init=0  period=sampPeriod  ownership=Locally owned and initialized  arith_type=Unsigned  n_bits=32  bin_pt=0  dbl_ovrd=off  block_type=fromreg
      settings: AttributesFormatString=From Register\n<< %<shared_memory_name> >>\n%<n_bits>\n%<period>\n%<bin_pt>\n%<arith_type>\n%<ownership>\n%<init>  infoedit="Register block that reads data to a shared memory register.  Delay of one sample period."  block_version=8.2.02
  D : Outport
  wires:
  From Register/1 -> D/1

== system ofdm_txrx_mimo_2x/Memory-mapped Registers/Tx Registers/Write-Only Register1 ==
  From Register : xbsIndex_r4/From Register  shared_memory_name='Tx_PreambleScaling_w'  init=16639.5904  period=sampPeriod  ownership=Locally owned and initialized  arith_type=Unsigned  n_bits=16  bin_pt=0  dbl_ovrd=off  block_type=fromreg
      settings: AttributesFormatString=From Register\n<< %<shared_memory_name> >>\n%<n_bits>\n%<period>\n%<bin_pt>\n%<arith_type>\n%<ownership>\n%<init>  infoedit="Register block that reads data to a shared memory register.  Delay of one sample period."  block_version=8.2.02
  D : Outport
  wires:
  From Register/1 -> D/1

== system ofdm_txrx_mimo_2x/Memory-mapped Registers/Tx Registers/Write-Only Register2 ==
  From Register : xbsIndex_r4/From Register  shared_memory_name='Tx_Pilots_Index1_w'  init=3735559  period=sampPeriod  ownership=Locally owned and initialized  arith_type=Unsigned  n_bits=32  bin_pt=0  dbl_ovrd=off  block_type=fromreg
      settings: AttributesFormatString=From Register\n<< %<shared_memory_name> >>\n%<n_bits>\n%<period>\n%<bin_pt>\n%<arith_type>\n%<ownership>\n%<init>  infoedit="Register block that reads data to a shared memory register.  Delay of one sample period."  block_version=8.2.02
  D : Outport
  wires:
  From Register/1 -> D/1

== system ofdm_txrx_mimo_2x/Memory-mapped Registers/Tx Registers/Write-Only Register3 ==
  From Register : xbsIndex_r4/From Register  shared_memory_name='Tx_Pilots_Index2_w'  init=2818069  period=sampPeriod  ownership=Locally owned and initialized  arith_type=Unsigned  n_bits=32  bin_pt=0  dbl_ovrd=off  block_type=fromreg
      settings: AttributesFormatString=From Register\n<< %<shared_memory_name> >>\n%<n_bits>\n%<period>\n%<bin_pt>\n%<arith_type>\n%<ownership>\n%<init>  infoedit="Register block that reads data to a shared memory register.  Delay of one sample period."  block_version=8.2.02
  D : Outport
  wires:
  From Register/1 -> D/1

== system ofdm_txrx_mimo_2x/Memory-mapped Registers/Tx Registers/Write-Only Register4 ==
  From Register : xbsIndex_r4/From Register  shared_memory_name='Tx_Pilots_Value1_w'  init=32768  period=sampPeriod  ownership=Locally owned and initialized  arith_type=Unsigned  n_bits=32  bin_pt=0  dbl_ovrd=off  block_type=fromreg
      settings: AttributesFormatString=From Register\n<< %<shared_memory_name> >>\n%<n_bits>\n%<period>\n%<bin_pt>\n%<arith_type>\n%<ownership>\n%<init>  infoedit="Register block that reads data to a shared memory register.  Delay of one sample period."  block_version=8.2.02
  D : Outport
  wires:
  From Register/1 -> D/1

== system ofdm_txrx_mimo_2x/Memory-mapped Registers/Tx Registers/Write-Only Register5 ==
  From Register : xbsIndex_r4/From Register  shared_memory_name='Tx_Pilots_Value2_w'  init=32767  period=sampPeriod  ownership=Locally owned and initialized  arith_type=Unsigned  n_bits=32  bin_pt=0  dbl_ovrd=off  block_type=fromreg
      settings: AttributesFormatString=From Register\n<< %<shared_memory_name> >>\n%<n_bits>\n%<period>\n%<bin_pt>\n%<arith_type>\n%<ownership>\n%<init>  infoedit="Register block that reads data to a shared memory register.  Delay of one sample period."  block_version=8.2.02
  D : Outport
  wires:
  From Register/1 -> D/1

== system ofdm_txrx_mimo_2x/Memory-mapped Registers/TxRx Registers/InterruptControl ==
  Reg_8b : Inport
  LSB+0 : xbsIndex_r4/Slice  nbits=1  boolean_output=on  mode=Lower Bit Location + Width  bit1=0  base1=MSB of Input  bit0=0  base0=LSB of Input  dbl_ovrd=off  block_type=slice
      settings: infoedit="Extracts a given range of bits from each input sample and presents it at the output.  The output type is ordinarily unsigned with binary point at zero, but can be Boolean when the slice is one bit wide.<P><P>Hardware notes: In hardware this block costs nothing."  block_version=VER_STRING_GOES_HERE
  LSB+1 : xbsIndex_r4/Slice  nbits=1  boolean_output=on  mode=Lower Bit Location + Width  bit1=0  base1=MSB of Input  bit0=1  base0=LSB of Input  dbl_ovrd=off  block_type=slice
      settings: infoedit="Extracts a given range of bits from each input sample and presents it at the output.  The output type is ordinarily unsigned with binary point at zero, but can be Boolean when the slice is one bit wide.<P><P>Hardware notes: In hardware this block costs nothing."  block_version=VER_STRING_GOES_HERE
  LSB+2 : xbsIndex_r4/Slice  nbits=1  boolean_output=off  mode=Lower Bit Location + Width  bit1=0  base1=MSB of Input  bit0=2  base0=LSB of Input  dbl_ovrd=off  block_type=slice
      settings: infoedit="Extracts a given range of bits from each input sample and presents it at the output.  The output type is ordinarily unsigned with binary point at zero, but can be Boolean when the slice is one bit wide.<P><P>Hardware notes: In hardware this block costs nothing."  block_version=VER_STRING_GOES_HERE
  LSB+3 : xbsIndex_r4/Slice  nbits=1  boolean_output=off  mode=Lower Bit Location + Width  bit1=0  base1=MSB of Input  bit0=3  base0=LSB of Input  dbl_ovrd=off  block_type=slice
      settings: infoedit="Extracts a given range of bits from each input sample and presents it at the output.  The output type is ordinarily unsigned with binary point at zero, but can be Boolean when the slice is one bit wide.<P><P>Hardware notes: In hardware this block costs nothing."  block_version=VER_STRING_GOES_HERE
  LSB+4 : xbsIndex_r4/Slice  nbits=1  boolean_output=off  mode=Lower Bit Location + Width  bit1=0  base1=MSB of Input  bit0=4  base0=LSB of Input  dbl_ovrd=off  block_type=slice
      settings: infoedit="Extracts a given range of bits from each input sample and presents it at the output.  The output type is ordinarily unsigned with binary point at zero, but can be Boolean when the slice is one bit wide.<P><P>Hardware notes: In hardware this block costs nothing."  block_version=VER_STRING_GOES_HERE
  LSB+5 : xbsIndex_r4/Slice  nbits=1  boolean_output=off  mode=Lower Bit Location + Width  bit1=0  base1=MSB of Input  bit0=5  base0=LSB of Input  dbl_ovrd=off  block_type=slice
      settings: infoedit="Extracts a given range of bits from each input sample and presents it at the output.  The output type is ordinarily unsigned with binary point at zero, but can be Boolean when the slice is one bit wide.<P><P>Hardware notes: In hardware this block costs nothing."  block_version=VER_STRING_GOES_HERE
  LSB+6 : xbsIndex_r4/Slice  nbits=1  boolean_output=off  mode=Lower Bit Location + Width  bit1=0  base1=MSB of Input  bit0=6  base0=LSB of Input  dbl_ovrd=off  block_type=slice
      settings: infoedit="Extracts a given range of bits from each input sample and presents it at the output.  The output type is ordinarily unsigned with binary point at zero, but can be Boolean when the slice is one bit wide.<P><P>Hardware notes: In hardware this block costs nothing."  block_version=VER_STRING_GOES_HERE
  LSB+7 : xbsIndex_r4/Slice  nbits=1  boolean_output=off  mode=Lower Bit Location + Width  bit1=0  base1=MSB of Input  bit0=7  base0=LSB of Input  dbl_ovrd=off  block_type=slice
      settings: infoedit="Extracts a given range of bits from each input sample and presents it at the output.  The output type is ordinarily unsigned with binary point at zero, but can be Boolean when the slice is one bit wide.<P><P>Hardware notes: In hardware this block costs nothing."  block_version=VER_STRING_GOES_HERE
  Posedge : Subsystem
  Posedge1 : Subsystem
  Posedge2 : Subsystem
  tag scope: intrCtrl_RxBadHeader_en=global, intrCtrl_RxBadPkt_en=global, intrCtrl_RxGoodHeader_en=global, intrCtrl_RxGoodPkt_en=global, intrCtrl_RxHeaderInterrupts_rst=global, intrCtrl_RxPktInterrupts_rst=global, intrCtrl_TxDone_en=global, intrCtrl_TxDone_rst=global
  wires:
  LSB+0/1 -> Posedge/1(D)
  LSB+1/1 -> Posedge1/1(D)
  Reg_8b/1 -> LSB+0/1, LSB+1/1, LSB+2/1, LSB+4/1, LSB+5/1, LSB+6/1, LSB+7/1, LSB+3/1
  Posedge/1(Q) -> [intrCtrl_RxPktInterrupts_rst]
  Posedge1/1(Q) -> [intrCtrl_RxHeaderInterrupts_rst]
  LSB+4/1 -> [intrCtrl_RxBadPkt_en]
  LSB+5/1 -> [intrCtrl_RxGoodHeader_en]
  LSB+6/1 -> [intrCtrl_RxBadHeader_en]
  LSB+7/1 -> [intrCtrl_TxDone_en]
  LSB+3/1 -> [intrCtrl_RxGoodPkt_en]
  LSB+2/1 -> Posedge2/1(D)
  Posedge2/1(Q) -> [intrCtrl_TxDone_rst]
